simulink model "TosAeb"  (R2021a)
settings: Solver=FixedStepDiscrete  StartTime=0.0  StopTime=inf  FixedStep=0.01  RelTol=1e-3
undefined symbols (not defined in the file or in its folder): ACCModSts, AccSts1, AEB_ObjectMovingOrientation, AEB_ObjectMovingState, CN_BTCActive, CN_CurrentGearR, CN_CurrentGearR_DHT, CN_PTCActive, CN_VDCActive, CN_VSC_EspOff, CN_VSC_FCW_AEB_Error, CN_VSC_FCW_AEB_NoDispay, CN_VSC_FCW_AEB_PerfmDgdtn, CN_VSC_FCW_AEB_TempUnAvlbl, CN_VSC_VehStandstill, CNaccelAvoidMax, CNaccelAvoidMin, CNbrakePedalPress, CNcomfortStrLeftAccelMax, CNcomfortStrLeftAccelMin, CNcomfortStrRightAccelMax, CNcomfortStrRightAccelMin, CNDrvDoorOpen, CNDrvModeEco, CNDrvModeNorm, CNDrvModeOther, CNDrvModeSport, CNDrvSBRreminder, CNNull, CNobjInvalidID, CNPassDoorOpen, CNPassSBRreminder, CNPEBSAccelNegInf, CNPEBSAccelNull, CNPEBSTimeInf, CNPEBSTimeNull, CNSTOPMAX, CNSTOPNULL, CNsubjWidth, CNSwtOFF, CNSwtON, CNTTCMAX, CNTTCNULL, CNVehConfigAEBCycNone, CNVehConfigAEBNone, CNVehConfigAEBPedNone, CNVehConfigFCWNone, CNVehConfigJANone, CollisionSeverity, FALSE, FCWSnvty, JACycObjCalcInfoDefaultParams, JAPedObjCalcInfoDefaultParams, JAVehObjCalcInfoDefaultParams, latCycObjCalcInfoDefaultParams, latPedObjCalcInfoDefaultParams, longObjCalcInfoDefaultParams, ModSts, movingStatus, ObjectType ... (+1112)
libraries: simulink x3031, AEBSFunctionLib x50, Ta_Library x33, AEBSStateMachineLib x23, Mylibrary x12, simulink_extras x7, ObjSelect_lib x3, dspstat3 x2
(part 85 of 102)

== system TosAeb/AEB_Objsel/TA_Processor/AEB_ObjSelect/TargetInfoCheck/Data_precess/TA_EffectCalculate/Obj_FilterFlgCalculate/Long_ObjFilter/OverlapCalculate/BoudingBoxCalculate_Else/Overlap_FlgCalculate/AbsPosn_InsectionFlg/SubjBoundingBox/SubjLUCoordinate ==
  inputSignals : Inport
  Add : Sum  Inputs=++--  OutDataTypeStr=single
  Add1 : Sum  Inputs=+++-  OutDataTypeStr=single
  Bus Selector : BusSelector  OutputSignals=dxPreRefPoint,dxFrontBumperToRearAxis,sincosCoef.cosCoef,subjWidth,sincosCoef.sinCoef,Long_Offset
  Bus Selector1 : BusSelector  OutputSignals=dyPreRefPoint,dxFrontBumperToRearAxis,sincosCoef.sinCoef,subjWidth,sincosCoef.cosCoef,Lat_Offset
  Gain : Gain  Gain=0.5  OutDataTypeStr=single
  Gain1 : Gain  Gain=0.5  OutDataTypeStr=single
  Product{,1..3} (4 alike) : Product  OutDataTypeStr=single
  dxPreLU : Outport
  dyPreLU : Outport  Port=2
  wires:
  Bus Selector/4 -> Product1/1  "<subjWidth>"
  Product3/1 -> Gain1/1
  Bus Selector1/1 -> Add1/1  "<dyPreRefPoint>"
  Gain1/1 -> Add1/3
  Bus Selector1/4 -> Product3/1  "<subjWidth>"
  Product2/1 -> Add1/2
  Bus Selector/3 -> Product/2  "<cosCoef>"
  Add1/1 -> dyPreLU/1
  Bus Selector/5 -> Product1/2  "<sinCoef>"
  Bus Selector/2 -> Product/1  "<dxFrontBumperToRearAxis>"
  Bus Selector1/2 -> Product2/1  "<dxFrontBumperToRearAxis>"
  inputSignals/1 -> Bus Selector1/1, Bus Selector/1
  Bus Selector1/5 -> Product3/2  "<cosCoef>"
  Bus Selector/1 -> Add/1  "<dxPreRefPoint>"
  Gain/1 -> Add/3
  Product1/1 -> Gain/1
  Product/1 -> Add/2
  Bus Selector1/3 -> Product2/2  "<sinCoef>"
  Bus Selector/6 -> Add/4  "<Long_Offset>"
  Add/1 -> dxPreLU/1
  Bus Selector1/6 -> Add1/4  "<Lat_Offset>"

== system TosAeb/AEB_Objsel/TA_Processor/AEB_ObjSelect/TargetInfoCheck/Data_precess/TA_EffectCalculate/Obj_FilterFlgCalculate/Long_ObjFilter/OverlapCalculate/BoudingBoxCalculate_Else/Overlap_FlgCalculate/AbsPosn_InsectionFlg/SubjBoundingBox/SubjRLCoordinate ==
  inputSignals : Inport
  Add6 : Sum  Inputs=+-+-  OutDataTypeStr=single
  Add7 : Sum  Inputs=+---  OutDataTypeStr=single
  Bus Selector6 : BusSelector  OutputSignals=dxPreRefPoint,dxRearBumperToRearAxis,sincosCoef.cosCoef,subjWidth,sincosCoef.sinCoef,Long_Offset
  Bus Selector7 : BusSelector  OutputSignals=dyPreRefPoint,dxRearBumperToRearAxis,sincosCoef.sinCoef,subjWidth,sincosCoef.cosCoef,Lat_Offset
  Gain6 : Gain  Gain=0.5  OutDataTypeStr=single
  Gain7 : Gain  Gain=0.5  OutDataTypeStr=single
  Product{12..15} (4 alike) : Product  OutDataTypeStr=single
  dxPreRL : Outport
  dyPreRL : Outport  Port=2
  wires:
  Product12/1 -> Add6/2
  Gain6/1 -> Add6/3
  Product13/1 -> Gain6/1
  Add6/1 -> dxPreRL/1
  Bus Selector6/4 -> Product13/1  "<subjWidth>"
  Bus Selector6/5 -> Product13/2  "<sinCoef>"
  Bus Selector6/1 -> Add6/1  "<dxPreRefPoint>"
  Bus Selector6/2 -> Product12/1  "<dxRearBumperToRearAxis>"
  Bus Selector6/3 -> Product12/2  "<cosCoef>"
  inputSignals/1 -> Bus Selector7/1, Bus Selector6/1
  Add7/1 -> dyPreRL/1
  Product14/1 -> Add7/2
  Gain7/1 -> Add7/3
  Bus Selector7/1 -> Add7/1  "<dyPreRefPoint>"
  Bus Selector7/2 -> Product14/1  "<dxRearBumperToRearAxis>"
  Bus Selector7/4 -> Product15/1  "<subjWidth>"
  Product15/1 -> Gain7/1
  Bus Selector7/5 -> Product15/2  "<cosCoef>"
  Bus Selector7/3 -> Product14/2  "<sinCoef>"
  Bus Selector6/6 -> Add6/4  "<Long_Offset>"
  Bus Selector7/6 -> Add7/4  "<Lat_Offset>"

== system TosAeb/AEB_Objsel/TA_Processor/AEB_ObjSelect/TargetInfoCheck/Data_precess/TA_EffectCalculate/Obj_FilterFlgCalculate/Long_ObjFilter/OverlapCalculate/BoudingBoxCalculate_Else/Overlap_FlgCalculate/AbsPosn_InsectionFlg/SubjBoundingBox/SubjRUCoordinate ==
  inputSignals : Inport
  Add4 : Sum  Inputs=+++-  OutDataTypeStr=single
  Add5 : Sum  Inputs=++--  OutDataTypeStr=single
  Bus Selector4 : BusSelector  OutputSignals=dxPreRefPoint,dxFrontBumperToRearAxis,sincosCoef.cosCoef,subjWidth,sincosCoef.sinCoef,Long_Offset
  Bus Selector5 : BusSelector  OutputSignals=dyPreRefPoint,dxFrontBumperToRearAxis,sincosCoef.sinCoef,subjWidth,sincosCoef.cosCoef,Lat_Offset
  Gain4 : Gain  Gain=0.5  OutDataTypeStr=single
  Gain5 : Gain  Gain=0.5  OutDataTypeStr=single
  Product{8..11} (4 alike) : Product  OutDataTypeStr=single
  dxPreRU : Outport
  dyPreRU : Outport  Port=2
  wires:
  Bus Selector4/4 -> Product9/1  "<subjWidth>"
  Bus Selector4/5 -> Product9/2  "<sinCoef>"
  Bus Selector4/3 -> Product8/2  "<cosCoef>"
  Bus Selector4/1 -> Add4/1  "<dxPreRefPoint>"
  inputSignals/1 -> Bus Selector5/1, Bus Selector4/1
  Bus Selector4/2 -> Product8/1  "<dxFrontBumperToRearAxis>"
  Product9/1 -> Gain4/1
  Bus Selector5/4 -> Product11/1  "<subjWidth>"
  Product10/1 -> Add5/2
  Product11/1 -> Gain5/1
  Add5/1 -> dyPreRU/1
  Gain5/1 -> Add5/3
  Bus Selector5/2 -> Product10/1  "<dxFrontBumperToRearAxis>"
  Bus Selector5/3 -> Product10/2  "<sinCoef>"
  Bus Selector5/5 -> Product11/2  "<cosCoef>"
  Add4/1 -> dxPreRU/1
  Gain4/1 -> Add4/3
  Product8/1 -> Add4/2
  Bus Selector5/1 -> Add5/1  "<dyPreRefPoint>"
  Bus Selector4/6 -> Add4/4  "<Long_Offset>"
  Bus Selector5/6 -> Add5/4  "<Lat_Offset>"

== system TosAeb/AEB_Objsel/TA_Processor/AEB_ObjSelect/TargetInfoCheck/Data_precess/TA_EffectCalculate/Obj_FilterFlgCalculate/Long_ObjFilter/OverlapCalculate/BoudingBoxCalculate_Else/Overlap_FlgCalculate/AbsPosn_InsectionFlg/SubjBoundingBox/Subsystem ==
  dxFrontBumperToRearAxis : Inport
  sincosCoef : Inport  Port=2
  Bus Selector : BusSelector  OutputSignals=sinCoef,cosCoef
  Product : Product
  Product1 : Product
  Lat_Offset : Outport
  Long_Offset : Outport  Port=2
  wires:
  Product/1 -> Lat_Offset/1  "Lat_Offset"
  dxFrontBumperToRearAxis/1 -> Product1/1, Product/1
  sincosCoef/1 -> Bus Selector/1
  Bus Selector/1 -> Product/2  "<sinCoef>"
  Bus Selector/2 -> Product1/2  "<cosCoef>"
  Product1/1 -> Long_Offset/1  "Long_Offset"

== system TosAeb/AEB_Objsel/TA_Processor/AEB_ObjSelect/TargetInfoCheck/Data_precess/TA_EffectCalculate/Obj_FilterFlgCalculate/Long_ObjFilter/OverlapCalculate/BoudingBoxCalculate_Else/PosnatTTI/LongitudinalMotionData/Subsystem1/Obj_InfoCal ==
  stop : Inport
  TA_ObjAccel : Inport  Port=2
  Subj_Ax : Inport  Port=3
  Subj_Ay : Inport  Port=4
  MappedAngle : Inport  Port=5
  Add : Sum
  Add1 : Sum
  Constant : Constant  Value=0  OutDataTypeStr=single
  Product : Product
  Product1 : Product
  Sin : Trigonometry  Operator=cos
  Sin1 : Trigonometry
  Switch : Switch  Criteria=u2 > Threshold
  Switch1 : Switch  Criteria=u2 > Threshold
  Obj_AbsAcclx : Outport
  Obj_AbsAccly : Outport  Port=2
  Ref_ax : Outport  Port=3
  Ref_ay : Outport  Port=4
  wires:
  Constant/1 -> Switch1/1
  Switch1/1 -> Ref_ay/1
  [Ta_ObjAccl] -> Switch/1
  Switch/1 -> Ref_ax/1
  stop/1 -> Switch1/2, Switch/2
  Add1/1 -> Obj_AbsAccly/1
  Subj_Ay/1 -> Add1/2
  Product1/1 -> Add1/1, Switch1/3
  Sin1/1 -> Product1/1
  [Ta_ObjAccl] -> Product1/2
  Subj_Ax/1 -> Add/2
  Add/1 -> Obj_AbsAcclx/1
  Product/1 -> Add/1, Switch/3
  TA_ObjAccel/1 -> Product/1, [Ta_ObjAccl]
  Sin/1 -> Product/2
  MappedAngle/1 -> Sin/1, Sin1/1

== system TosAeb/AEB_Objsel/TA_Processor/AEB_ObjSelect/TargetInfoCheck/Data_precess/TA_EffectCalculate/Obj_FilterFlgCalculate/Long_ObjFilter/OverlapCalculate/BoudingBoxCalculate_Else/PosnatTTI/LongitudinalMotionData/Subsystem1/Subj_AyCal ==
  Subj_Vx : Inport
  Subj_Vy : Inport  Port=2
  ACC_x : Inport  Port=3
  Add : Sum  Inputs=-+
  Atan2 : Trigonometry  Operator=atan2
  Compare To Constant : simulink/Logic and Bit Operations/Compare To Constant  relop===  const=0  OutDataTypeStr=boolean
  Constant : Constant  Value=pi/2  OutDataTypeStr=single
  Constant1 : Constant  Value=0  OutDataTypeStr=single
  Constant2 : Constant  Value=0.01  OutDataTypeStr=single
  Delay : Delay
  Divide : Product  Inputs=*/
  MapToAngularInterval : Ta_Library/MapToAngularInterval  SystemSampleTime=-1  Latency=0  AutoFrameSizeCalculation=off
  Product : Product
  Sin : Trigonometry  Operator=tan
  Switch : Switch  Criteria=u2 > Threshold
  Terminator : Terminator
  Subj_Ay : Outport
  wires:
  Divide/1 -> Terminator/1
  Constant2/1 -> Divide/2
  Add/1 -> Divide/1
  Delay/1 -> Add/1
  Product/1 -> Subj_Ay/1
  ACC_x/1 -> Product/1
  Switch/1 -> Product/2
  Constant1/1 -> Switch/1
  Compare To Constant/1 -> Switch/2
  Sin/1 -> Compare To Constant/1, Switch/3
  MapToAngularInterval/1 -> Sin/1
  Atan2/1 -> MapToAngularInterval/1
  Constant/1 -> MapToAngularInterval/2
  Subj_Vy/1 -> Atan2/1, Delay/1, Add/2
  Subj_Vx/1 -> Atan2/2

== system TosAeb/AEB_Objsel/TA_Processor/AEB_ObjSelect/TargetInfoCheck/Data_precess/TA_EffectCalculate/Obj_FilterFlgCalculate/Long_ObjFilter/OverlapCalculate/BoudingBoxCalculate_Else/PosnatTTI/PredictHostPositionAtTTR/CalculateHeading/CheckSpeed ==
  note: Threshold for detectable host speed.
  note: 0.2m/s
  x : Inport
  y : Inport  Port=2
  LatSpdThresholdNonVeh1 : Constant  Value=SpdThdInAsyEvlrCritEve
  Product : Product
  Product1 : Product
  Product2 : Product
  Relational Operator : RelationalOperator  Operator=>  OutDataTypeStr=boolean  RndMeth=Nearest
  Subtract : Sum
  SpeedValid : Outport
  wires:
  y/1 -> Product/1, Product/2
  Subtract/1 -> Relational Operator/1  "VelocitySquared"
  Product1/1 -> Subtract/1
  Product/1 -> Subtract/2
  x/1 -> Product1/2, Product1/1
  LatSpdThresholdNonVeh1/1 -> Product2/1, Product2/2
  Product2/1 -> Relational Operator/2
  Relational Operator/1 -> SpeedValid/1  "SpeedValid"

== system TosAeb/AEB_Objsel/TA_Processor/AEB_ObjSelect/TargetInfoCheck/Data_precess/TA_EffectCalculate/Obj_FilterFlgCalculate/Long_ObjFilter/OverlapCalculate/BoudingBoxCalculate_Else/PosnatTTI/PredictHostPositionAtTTR/CalculateHeading/ExtractVelocitiesAtStartOfSegments ==
  PathEstimn : Inport
  Assignment : Assignment  OutputInitialize=Specify size for each dimension in table  IndexOptions=Index vector (port)  Indices=1  OutputSizes=4
  Assignment1 : Assignment  OutputInitialize=Specify size for each dimension in table  IndexOptions=Index vector (port)  Indices=1  OutputSizes=4
  Assignment2 : Assignment  OutputInitialize=Specify size for each dimension in table  IndexOptions=Index vector (port)  Indices=1  OutputSizes=4
  CheckSpeed : Subsystem
  ExtractVelocityVectorFirstPoint : Subsystem
  For Iterator : ForIterator  ResetStates=reset  IterationLimit=4  IterationVariableDataType=int8
  Selector : Selector  InputPortWidth=4  IndexOptions=Index vector (port)  Indices=1  OutputSizes=1
  SpeedValid : Outport
  SegmentVelocitiesX : Outport  Port=2
  SegmentVelocitiesY : Outport  Port=3
  wires:
  Assignment2/1 -> SegmentVelocitiesY/1  "SegmentVelocitiesY"
  Assignment1/1 -> SegmentVelocitiesX/1  "SegmentVelocitiesX"
  Assignment/1 -> SpeedValid/1  "SpeedValid"
  CheckSpeed/1(SpeedValid) -> Assignment/1
  For Iterator/1 -> Selector/2, Assignment1/2, Assignment2/2, Assignment/2
  PathEstimn/1 -> Selector/1
  ExtractVelocityVectorFirstPoint/2(dy) -> Assignment2/1, CheckSpeed/2(y)
  ExtractVelocityVectorFirstPoint/1(dx) -> Assignment1/1, CheckSpeed/1(x)
  Selector/1 -> ExtractVelocityVectorFirstPoint/1(Segment)  "Segment"

== system TosAeb/AEB_Objsel/TA_Processor/AEB_ObjSelect/TargetInfoCheck/Data_precess/TA_EffectCalculate/Obj_FilterFlgCalculate/Long_ObjFilter/OverlapCalculate/BoudingBoxCalculate_Else/PosnatTTI/PredictHostPositionAtTTR/SegmentComponents/SafeDivide ==
  Numerator : Inport  OutDataTypeStr=DataType
      settings: AttributesFormatString=DT=%<OutDataTypeStr>
  Denominator : Inport  Port=2  OutDataTypeStr=DataType
      settings: AttributesFormatString=DT=%<OutDataTypeStr>
  SafeDivide : MATLAB Function
  Quotient : Outport  OutDataTypeStr=DataType
      settings: AttributesFormatString=DT=%<OutDataTypeStr>
  wires:
  SafeDivide/1(Quotient) -> Quotient/1
  Denominator/1 -> SafeDivide/2(Denominator)  "Denominator"
  Numerator/1 -> SafeDivide/1(Numerator)  "Numerator"

== system TosAeb/AEB_Objsel/TA_Processor/AEB_ObjSelect/TargetInfoCheck/Data_precess/TA_EffectCalculate/Obj_FilterFlgCalculate/Long_ObjFilter/OverlapCalculate/BoudingBoxCalculate_Else/PosnatTTI/PredictHostPositionAtTTR/SelectSegment/ForEachSegmentInPahEstimn ==
  PahEstimn : Inport  OutDataTypeStr=Bus: PahEstimn
  Time : Inport  Port=2
  Assignment1 : Assignment  OutputInitialize=Specify size for each dimension in table  IndexOptions=Index vector (port)  Indices=1  OutputSizes=4
  Bus Selector : BusSelector  OutputSignals=TiAry
  For Iterator : ForIterator  ResetStates=reset  IterationSource=external  IterationVariableDataType=int8
      settings: AttributesFormatString=DT=%<IterationVariableDataType>\nResetStates=%<ResetStates>
  Logical Operator : Logic  AllPortsSameDT=off  OutDataTypeStr=boolean
  Min : MinMax  Function=max  OutDataTypeStr=single
  Min1 : MinMax  OutDataTypeStr=single
  Relational Operator : RelationalOperator  Operator=>  OutDataTypeStr=boolean  RndMeth=Nearest  InputSameDT=on
      settings: AttributesFormatString=InputSameDT=%<InputSameDT>
  Relational Operator1 : RelationalOperator  Operator=<=  OutDataTypeStr=boolean  RndMeth=Nearest  InputSameDT=on
      settings: AttributesFormatString=InputSameDT=%<InputSameDT>
  Selector2 : Selector  IndexOptions=Index vector (port)  Indices=[1 3]  OutputSizes=1
  Width : Width  DataType=int8
  TimeWithinSegment : Outport
  wires:
  Min/1 -> Relational Operator1/2
  Bus Selector/1 -> Min/1, Min1/1  "<TiAry>"
  Time/1 -> Relational Operator1/1, [Time]
  Min1/1 -> Relational Operator/2
  [Time] -> Relational Operator/1
  Relational Operator1/1 -> Logical Operator/1
  Relational Operator/1 -> Logical Operator/2
  [CurrentIndex] -> Selector2/2
  [CurrentIndex] -> Assignment1/2
  Logical Operator/1 -> Assignment1/1  "TimeWithinSegment"
  Assignment1/1 -> TimeWithinSegment/1
  PahEstimn/1 -> Selector2/1, [PahEstimn]
  Selector2/1 -> Bus Selector/1
  Width/1 -> For Iterator/1
  [PahEstimn] -> Width/1
  For Iterator/1 -> [CurrentIndex]

== system TosAeb/AEB_Objsel/TA_Processor/AEB_ObjSelect/TargetInfoCheck/Data_precess/TA_EffectCalculate/Obj_FilterFlgCalculate/Long_ObjFilter/TTR_Calculate/CalculateTTRAndTTM_else/LongitudinalTTRComputer/CalculateTTI/HostAndObjectMoving/CheckIfSolutionExists ==
  note: 相对加速度
  note: 相对速度
  note: This subsystem checks if there is a solution to the motion equation ARel*t^2/2 + VRel*t + EdgeDistance = 0.
      |
      | The cases with positive and negative ARel are handled separately.
      | If ARel == 0, there is always a solution if VRel ~= 0.
      | The case VRel == 0 and ARel == 0 is handled outside this subsystem.
  note: Same zero threshold as in the
      | quadratic equation solver.
  note: Solution exists if ARel == 0.
  EdgeDistance : Inport
  VRel : Inport  Port=2
  ARel : Inport  Port=3
  Compare To Zero : Subsystem  mask(Compare To Zero){relop=>, OutDataTypeStr=boolean, ZeroCross=on}
      mask prompts: relop "Simulink:masks:Operator_MP"; OutDataTypeStr "Simulink:masks:OutputDataType_MP"; ZeroCross "Simulink:masks:EnableZerocrossingDetection_MP"
      mask help: Simulink:masks:CompareToZero_MD
      mask init: same as TosAeb/AEB_Objsel/TA_Processor/AEB_ObjSelect/TargetInfoCheck/Data_precess/TA_EffectCalculate/Obj_FilterFlgCalculate/Long_ObjFilter/TTR_Calculate/CalculateTTRAndTTM_else/ModifyTTRAtSameSpeedAndAcceleration/Compare To Zero
      mask TypeOptions relop: == | ~= | < | <= | >= | >
      mask TypeOptions OutDataTypeStr: Simulink:masks:uint8 | Simulink:masks:boolean
      mask Callback ZeroCross: sl('zeroCrossEnable', gcbh);
      mask Display: disp([relop,' 0'])
  Compare To Zero1 : Subsystem  mask(Compare To Zero){relop=<, OutDataTypeStr=boolean, ZeroCross=on}
      mask prompts: relop "Simulink:masks:Operator_MP"; OutDataTypeStr "Simulink:masks:OutputDataType_MP"; ZeroCross "Simulink:masks:EnableZerocrossingDetection_MP"
      mask help: Simulink:masks:CompareToZero_MD
      mask init: same as TosAeb/AEB_Objsel/TA_Processor/AEB_ObjSelect/TargetInfoCheck/Data_precess/TA_EffectCalculate/Obj_FilterFlgCalculate/Long_ObjFilter/TTR_Calculate/CalculateTTRAndTTM_else/ModifyTTRAtSameSpeedAndAcceleration/Compare To Zero
      mask TypeOptions relop: == | ~= | < | <= | >= | >
      mask TypeOptions OutDataTypeStr: Simulink:masks:uint8 | Simulink:masks:boolean
      mask Callback ZeroCross: sl('zeroCrossEnable', gcbh);
      mask Display: disp([relop,' 0'])
  Constant : Constant  Value=false  OutDataTypeStr=boolean
  Gain : Gain  Gain=2
  LimitedSafeDivide : Subsystem
  Logop_1 : Logic  AllPortsSameDT=off  OutDataTypeStr=boolean
  Logop_2 : Logic  AllPortsSameDT=off  OutDataTypeStr=boolean
  Logop_3 : Logic  Operator=OR  AllPortsSameDT=off  OutDataTypeStr=boolean
  Relop_1 : RelationalOperator  Operator=>  OutDataTypeStr=boolean  RndMeth=Nearest
  Relop_2 : RelationalOperator  Operator=<  OutDataTypeStr=boolean  RndMeth=Nearest
  SizeChecker : Subsystem  mask(Size checker){UseInput=off, Limit=1e-2, DataTypeIn=single, Operator=>}
      mask prompts: UseInput "Take the limit as an input"; DataTypeIn "Data type:"; Operator "Relational operator:"
      mask help: The block tests the absolute value of the supplied signal against a specified value using the selected operator. If the signal conforms to the test, true is returned; else, false is returned. The limit can either be set in the mask or as an external signal.
      mask init: same as TosAeb/AEB_Objsel/TA_Processor/AEB_ObjSelect/TargetInfoCheck/Data_precess/TA_EffectCalculate/Obj_FilterFlgCalculate/Long_ObjFilter/TTR_Calculate/CalculateTTRAndTTM_else/ModifyTTRAtSameSpeedAndAcceleration/SizeChecker
      settings: AttributesFormatString=DT=%<DataTypeIn>
      mask Help: The block tests the absolute value of the supplied signal against a specified value using the selected operator. If the signal conforms to the test, true is returned; else, false is returned. The limit can either be set in the mask or as an external signal.
      mask Callback UseInput:
      | % Author: Jakob Andersson (jande573)
      | 
      | % Enable and disable the box for the limit variable depending on the status of the checkbox
      | if strcmp(get_param(gcb,'UseInput'),'on')
      |     % Disable the box
      |     set_param(gcb,'MaskEnables',{'on','off','on','on'}); % One setting for each parameter in this mask; needs to be updated if the parameters are changed
      | else
      |     % Enable the box
      |     set_param(gcb,'MaskEnables',{'on','on','on','on'}); % One setting for each parameter in this mask; needs to be updated if the parameters are changed
      | end
      mask Callback Limit:
      | % Author: Jakob Andersson (jande573)
      | 
      | % Define the default value to apply if the entered value is disallowed
      | default='1e-6';
      | 
      | % Evaluate the entered value
      | val=eval(get_param(gcb,'Limit'));
      | 
      | % Test the entered value and revert to the default if necessary
      | if isnumeric(val)==false
      |     % Set the default if the entered value did not evaluate to a numeric
      |     set_param(gcb,'Limit',default);
      |     errordlg('The limit must be numeric. The default value will be used instead.');
      | elseif val<0
      |     % Set the default if the entered value is negative
      |     set_param(gcb,'Limit',default);
      |     errordlg('The limit must be positive since it is compared to an absolute value. The default value will be used instead.');
      | end
      mask TypeOptions Operator:
      | Relational
      | Operator/Operator
      mask Callback Operator:
      | % Author: Jakob Andersson (jande573)
      | 
      | % Define the default value to apply if the entered value is disallowed
      | EnabledOperators={'==','~=','<','<=','>=','>'};
      | default='>';
      | 
      | % Evaluate the selected operator and determine if it is ok
      | ok=any(strcmp(get_param(gcb,'Operator'),EnabledOperators));
      | 
      | % Revert the operator if it is disabled
      | if ~ok
      |     % Set the default if the entered value did not evaluate to a numeric
      |     set_param(gcb,'Operator',default);
      |     errordlg('The selected operator is disabled. The default operator will be used instead.');
      | end
      mask Display:
      | % Display the mask for this block
      | % Author: Jakob Andersson (jande573)
      | 
      | % Transform the operator to TeX
      | switch Operator
      |     case '=='
      |         op='=';
      |     case '~='
      |         op='\neq';
      |     case '<='
      |         op='\leq';
      |     case '>='
      |         op='\geq';
      |     otherwise
      |         op=Operator;
      | end
      | 
      | % Display the correct mask depending on the number of inputs
      | if strcmp(UseInput,'on')
      |     % Display the block logic and the set limit
      |     disp(horzcat('|u| ',op,' a'),'texmode','on');
      | 
      |     % Display the port title
      |     port_label('input',1,'u');
      |     port_label('input',2,'a');
      | else
      |     % Display the block logic and the set limit
      |     disp(horzcat('|u| ',op,' ',num2str(Limit)),'texmode','on');
      | 
      |     % Display the port title
      |     port_label('input',1,'u');
      | end
  Square : Math  Operator=square  SignedPower=on  SaturateOnIntegerOverflow=on
  Switch : Switch  Criteria=u2 ~= 0
  NoSolution : Outport
  wires:
  Constant/1 -> Switch/3  "False"
  Switch/1 -> NoSolution/1  "NoSolution"
  SizeChecker/1(TestOk) -> Switch/2  "ARelIsDetectable"
  VRel/1 -> Square/1
  ARel/1 -> Compare To Zero/1(u), Compare To Zero1/1(u), SizeChecker/1(Signal), Gain/1
  EdgeDistance/1 -> Relop_1/1, Relop_2/1
  Square/1 -> LimitedSafeDivide/1(Numerator)
  Gain/1 -> LimitedSafeDivide/2(Denominator)
  LimitedSafeDivide/1(LimitedQuotient) -> Relop_1/2, Relop_2/2
  Compare To Zero/1(y) -> Logop_1/2  "PositiveARel"
  Relop_1/1 -> Logop_1/1
  Compare To Zero1/1(y) -> Logop_2/2  "NegativeARel"
  Logop_1/1 -> Logop_3/1  "NoSolutionPositiveARel"
  Logop_2/1 -> Logop_3/2  "NoSolutionNegativeARel"
  Logop_3/1 -> Switch/1  "NoSolutionCalculatedUsingARel"
  [BelowLimit] -> Logop_2/1
  Relop_2/1 -> [BelowLimit]

== system TosAeb/AEB_Objsel/TA_Processor/AEB_ObjSelect/TargetInfoCheck/Data_precess/TA_EffectCalculate/Obj_FilterFlgCalculate/Long_ObjFilter/TTR_Calculate/CalculateTTRAndTTM_else/LongitudinalTTRComputer/CalculateTTI/HostAndObjectMoving/CheckIfSolutionExists1 ==
  note: This subsystem checks if there is a solution to the motion equation ARel*t^2/2 + VRel*t + EdgeDistance = 0.
      |
      | The cases with positive and negative ARel are handled separately.
      | If ARel == 0, there is always a solution if VRel ~= 0.
      | The case VRel == 0 and ARel == 0 is handled outside this subsystem.
  note: Same zero threshold as in the
      | quadratic equation solver.
  note: Solution exists if ARel == 0.
  EdgeDistance : Inport
  VRel : Inport  Port=2
  ARel : Inport  Port=3
  Compare To Zero : Subsystem  mask(Compare To Zero){relop=>, OutDataTypeStr=boolean, ZeroCross=on}
      mask prompts: relop "Simulink:masks:Operator_MP"; OutDataTypeStr "Simulink:masks:OutputDataType_MP"; ZeroCross "Simulink:masks:EnableZerocrossingDetection_MP"
      mask help: Simulink:masks:CompareToZero_MD
      mask init: same as TosAeb/AEB_Objsel/TA_Processor/AEB_ObjSelect/TargetInfoCheck/Data_precess/TA_EffectCalculate/Obj_FilterFlgCalculate/Long_ObjFilter/TTR_Calculate/CalculateTTRAndTTM_else/ModifyTTRAtSameSpeedAndAcceleration/Compare To Zero
      mask TypeOptions relop: == | ~= | < | <= | >= | >
      mask TypeOptions OutDataTypeStr: Simulink:masks:uint8 | Simulink:masks:boolean
      mask Callback ZeroCross: sl('zeroCrossEnable', gcbh);
      mask Display: disp([relop,' 0'])
  Compare To Zero1 : Subsystem  mask(Compare To Zero){relop=<, OutDataTypeStr=boolean, ZeroCross=on}
      mask prompts: relop "Simulink:masks:Operator_MP"; OutDataTypeStr "Simulink:masks:OutputDataType_MP"; ZeroCross "Simulink:masks:EnableZerocrossingDetection_MP"
      mask help: Simulink:masks:CompareToZero_MD
      mask init: same as TosAeb/AEB_Objsel/TA_Processor/AEB_ObjSelect/TargetInfoCheck/Data_precess/TA_EffectCalculate/Obj_FilterFlgCalculate/Long_ObjFilter/TTR_Calculate/CalculateTTRAndTTM_else/ModifyTTRAtSameSpeedAndAcceleration/Compare To Zero
      mask TypeOptions relop: == | ~= | < | <= | >= | >
      mask TypeOptions OutDataTypeStr: Simulink:masks:uint8 | Simulink:masks:boolean
      mask Callback ZeroCross: sl('zeroCrossEnable', gcbh);
      mask Display: disp([relop,' 0'])
  Constant : Constant  Value=false  OutDataTypeStr=boolean
  Gain : Gain  Gain=2
  LimitedSafeDivide : Subsystem
  Logop_1 : Logic  AllPortsSameDT=off  OutDataTypeStr=boolean
  Logop_2 : Logic  AllPortsSameDT=off  OutDataTypeStr=boolean
  Logop_3 : Logic  Operator=OR  AllPortsSameDT=off  OutDataTypeStr=boolean
  Relop_1 : RelationalOperator  Operator=>  OutDataTypeStr=boolean  RndMeth=Nearest
  Relop_2 : RelationalOperator  Operator=<  OutDataTypeStr=boolean  RndMeth=Nearest
  SizeChecker : Subsystem  mask(Size checker){UseInput=off, Limit=1e-2, DataTypeIn=single, Operator=>}
      mask prompts: UseInput "Take the limit as an input"; DataTypeIn "Data type:"; Operator "Relational operator:"
      mask help: The block tests the absolute value of the supplied signal against a specified value using the selected operator. If the signal conforms to the test, true is returned; else, false is returned. The limit can either be set in the mask or as an external signal.
      mask init: same as TosAeb/AEB_Objsel/TA_Processor/AEB_ObjSelect/TargetInfoCheck/Data_precess/TA_EffectCalculate/Obj_FilterFlgCalculate/Long_ObjFilter/TTR_Calculate/CalculateTTRAndTTM_else/ModifyTTRAtSameSpeedAndAcceleration/SizeChecker
      settings: AttributesFormatString=DT=%<DataTypeIn>
      mask Help: The block tests the absolute value of the supplied signal against a specified value using the selected operator. If the signal conforms to the test, true is returned; else, false is returned. The limit can either be set in the mask or as an external signal.
      mask Callback UseInput:
      | % Author: Jakob Andersson (jande573)
      | 
      | % Enable and disable the box for the limit variable depending on the status of the checkbox
      | if strcmp(get_param(gcb,'UseInput'),'on')
      |     % Disable the box
      |     set_param(gcb,'MaskEnables',{'on','off','on','on'}); % One setting for each parameter in this mask; needs to be updated if the parameters are changed
      | else
      |     % Enable the box
      |     set_param(gcb,'MaskEnables',{'on','on','on','on'}); % One setting for each parameter in this mask; needs to be updated if the parameters are changed
      | end
      mask Callback Limit:
      | % Author: Jakob Andersson (jande573)
      | 
      | % Define the default value to apply if the entered value is disallowed
      | default='1e-6';
      | 
      | % Evaluate the entered value
      | val=eval(get_param(gcb,'Limit'));
      | 
      | % Test the entered value and revert to the default if necessary
      | if isnumeric(val)==false
      |     % Set the default if the entered value did not evaluate to a numeric
      |     set_param(gcb,'Limit',default);
      |     errordlg('The limit must be numeric. The default value will be used instead.');
      | elseif val<0
      |     % Set the default if the entered value is negative
      |     set_param(gcb,'Limit',default);
      |     errordlg('The limit must be positive since it is compared to an absolute value. The default value will be used instead.');
      | end
      mask TypeOptions Operator:
      | Relational
      | Operator/Operator
      mask Callback Operator:
      | % Author: Jakob Andersson (jande573)
      | 
      | % Define the default value to apply if the entered value is disallowed
      | EnabledOperators={'==','~=','<','<=','>=','>'};
      | default='>';
      | 
      | % Evaluate the selected operator and determine if it is ok
      | ok=any(strcmp(get_param(gcb,'Operator'),EnabledOperators));
      | 
      | % Revert the operator if it is disabled
      | if ~ok
      |     % Set the default if the entered value did not evaluate to a numeric
      |     set_param(gcb,'Operator',default);
      |     errordlg('The selected operator is disabled. The default operator will be used instead.');
      | end
      mask Display:
      | % Display the mask for this block
      | % Author: Jakob Andersson (jande573)
      | 
      | % Transform the operator to TeX
      | switch Operator
      |     case '=='
      |         op='=';
      |     case '~='
      |         op='\neq';
      |     case '<='
      |         op='\leq';
      |     case '>='
      |         op='\geq';
      |     otherwise
      |         op=Operator;
      | end
      | 
      | % Display the correct mask depending on the number of inputs
      | if strcmp(UseInput,'on')
      |     % Display the block logic and the set limit
      |     disp(horzcat('|u| ',op,' a'),'texmode','on');
      | 
      |     % Display the port title
      |     port_label('input',1,'u');
      |     port_label('input',2,'a');
      | else
      |     % Display the block logic and the set limit
      |     disp(horzcat('|u| ',op,' ',num2str(Limit)),'texmode','on');
      | 
      |     % Display the port title
      |     port_label('input',1,'u');
      | end
  Square : Math  Operator=square  SignedPower=on  SaturateOnIntegerOverflow=on
  Switch : Switch  Criteria=u2 ~= 0
  NoSolution : Outport
  wires:
  Constant/1 -> Switch/3  "False"
  Switch/1 -> NoSolution/1  "NoSolution"
  SizeChecker/1(TestOk) -> Switch/2  "ARelIsDetectable"
  VRel/1 -> Square/1
  ARel/1 -> Compare To Zero/1(u), Compare To Zero1/1(u), SizeChecker/1(Signal), Gain/1
  EdgeDistance/1 -> Relop_1/1, Relop_2/1
  Square/1 -> LimitedSafeDivide/1(Numerator)
  Gain/1 -> LimitedSafeDivide/2(Denominator)
  LimitedSafeDivide/1(LimitedQuotient) -> Relop_1/2, Relop_2/2
  Compare To Zero/1(y) -> Logop_1/2  "PositiveARel"
  Relop_1/1 -> Logop_1/1
  Compare To Zero1/1(y) -> Logop_2/2  "NegativeARel"
  Logop_1/1 -> Logop_3/1  "NoSolutionPositiveARel"
  Logop_2/1 -> Logop_3/2  "NoSolutionNegativeARel"
  Logop_3/1 -> Switch/1  "NoSolutionCalculatedUsingARel"
  [BelowLimit] -> Logop_2/1
  Relop_2/1 -> [BelowLimit]

== system TosAeb/AEB_Objsel/TA_Processor/AEB_ObjSelect/TargetInfoCheck/Data_precess/TA_EffectCalculate/Obj_FilterFlgCalculate/Long_ObjFilter/TTR_Calculate/CalculateTTRAndTTM_else/LongitudinalTTRComputer/CalculateTTI/HostAndObjectMoving/TimeToIntersectionComputer ==
  note: 求解一元二次方程，并判断是否存在解、
      | 依据位置关系进行判断输出TTI数值
  note: 100
  note: -100
  note: a*t^2/2 + v*t + x = 0
  VRel : Inport  OutDataTypeStr=single
      settings: AttributesFormatString=DT=%<OutDataTypeStr>
  ARel : Inport  Port=2  OutDataTypeStr=single
      settings: AttributesFormatString=DT=%<OutDataTypeStr>
  Posn : Inport  Port=3  OutDataTypeStr=single
      settings: AttributesFormatString=DT=%<OutDataTypeStr>
  AEB_State : Inport  Port=4
  Abs : Abs
  Abs1 : Abs
  Compare To Constant : simulink/Logic and Bit Operations/Compare To Constant  relop=<=  const=0.25  OutDataTypeStr=boolean
  Compare To Constant1 : simulink/Logic and Bit Operations/Compare To Constant  relop===  const=0  OutDataTypeStr=boolean
  Compare To Constant10 : simulink/Logic and Bit Operations/Compare To Constant  relop===  const=0  OutDataTypeStr=boolean
  Compare To Constant2 : simulink/Logic and Bit Operations/Compare To Constant  relop=~=  const=0  OutDataTypeStr=boolean
  Compare To Constant3 : simulink/Logic and Bit Operations/Compare To Constant  relop=<=  const=0.2  OutDataTypeStr=boolean
  Compare To Constant4 : simulink/Logic and Bit Operations/Compare To Constant  relop=~=  const=100  OutDataTypeStr=boolean
  Compare To Constant5 : simulink/Logic and Bit Operations/Compare To Constant  relop=~=  const=-100  OutDataTypeStr=boolean
  Compare To Constant7 : simulink/Logic and Bit Operations/Compare To Constant  relop=~=  const=0  OutDataTypeStr=boolean
  Compare To Constant8 : simulink/Logic and Bit Operations/Compare To Constant  relop=<=  const=6  OutDataTypeStr=boolean
  Compare To Constant9 : simulink/Logic and Bit Operations/Compare To Constant  relop=<=  const=6  OutDataTypeStr=boolean
  Compare To Zero : Subsystem  mask(Compare To Zero){relop=<, OutDataTypeStr=boolean, ZeroCross=on}
      mask prompts: relop "Simulink:masks:Operator_MP"; OutDataTypeStr "Simulink:masks:OutputDataType_MP"; ZeroCross "Simulink:masks:EnableZerocrossingDetection_MP"
      mask help: Simulink:masks:CompareToZero_MD
      mask init: same as TosAeb/AEB_Objsel/TA_Processor/AEB_ObjSelect/TargetInfoCheck/Data_precess/TA_EffectCalculate/Obj_FilterFlgCalculate/Long_ObjFilter/TTR_Calculate/CalculateTTRAndTTM_else/ModifyTTRAtSameSpeedAndAcceleration/Compare To Zero
      mask TypeOptions relop: == | ~= | < | <= | >= | >
      mask TypeOptions OutDataTypeStr: Simulink:masks:uint8 | Simulink:masks:boolean
      mask Callback ZeroCross: sl('zeroCrossEnable', gcbh);
      mask Display: disp([relop,' 0'])
  Constant : Constant  OutDataTypeStr=single
  Constant1 : Constant  Value=P_TA_TiLowrLimInAsyEvlrCritEve  OutDataTypeStr=single
      settings: AttributesFormatString=DT=%<OutDataTypeStr>
  Constant3 : Constant  Value=P_TA_TiUpprLimInAsyEvlrCritEve  OutDataTypeStr=single
      settings: AttributesFormatString=DT=%<OutDataTypeStr>
  Delay : Delay  DelayLength=5
  Divide : Product  Inputs=*/
  Gain : Gain  Gain=0.5
  Logical Operator : Logic  Operator=OR  Inputs=3  AllPortsSameDT=off  OutDataTypeStr=boolean
  Logical Operator1 : Logic  Inputs=3  AllPortsSameDT=off  OutDataTypeStr=boolean
  Logical Operator2 : Logic  AllPortsSameDT=off  OutDataTypeStr=boolean
  Logical Operator3 : Logic  Inputs=3  AllPortsSameDT=off  OutDataTypeStr=boolean
  QuadraticEquationSolver : MATLAB Function  TreatAsAtomicUnit=on  RTWFileName=QuadraticEquationSolverNew  mask(Quadratic equation solver){ZeroThreshold=single(1e-2)}
      mask prompts: ZeroThreshold "Zero threshold:"
      mask help:
      | This block solves a quadratic polynomial equation.
      | Both solutions are returned in a bus.
      | If the equation lacks real roots then zeros are returned,
      | along with a flag indicating that no solutions where found.
      settings: RTWFcnNameOpts=Use subsystem name
  Relational Operator : RelationalOperator  Operator=<=  OutDataTypeStr=boolean  RndMeth=Simplest
  SolutionChooser : Subsystem
  Switch : Switch  Criteria=u2 > Threshold
  Switch1 : Switch  Criteria=u2 ~= 0  InputSameDT=on
      settings: AttributesFormatString=InputSameDT=%<InputSameDT>
  Switch2 : Switch  Criteria=u2 ~= 0  InputSameDT=on
      settings: AttributesFormatString=InputSameDT=%<InputSameDT>
  Switch3 : Switch  Criteria=u2 > Threshold
  Switch4 : Switch  Criteria=u2 > Threshold
  Terminator1 : Terminator
  Unit Delay : UnitDelay  SampleTime=-1  HasFrameUpgradeWarning=on
  TimeToIntersection : Outport  OutDataTypeStr=single
      settings: AttributesFormatString=DT=%<OutDataTypeStr>
  wires:
  Switch1/1 -> Switch2/3  "DefaultTTI"
  Constant1/1 -> Switch1/1  "DefaultNegativeTTI"
  Compare To Zero/1(y) -> Switch1/2
  SolutionChooser/1(Solution) -> Switch2/1
  QuadraticEquationSolver/2(SolutionFound) -> [Solution], Switch2/2
  QuadraticEquationSolver/1(x) -> SolutionChooser/1(Solutions)
  Posn/1 -> [B], Divide/1, QuadraticEquationSolver/3(c), Compare To Zero/1(u)  "Posn"
  VRel/1 -> QuadraticEquationSolver/2(b), [VRel]  "VRel"
  Switch2/1 -> Switch/3  "TimeToIntersection"
  Constant3/1 -> Switch1/3  "DefaultPositiveTTI"
  Gain/1 -> QuadraticEquationSolver/1(a)  "ARelHalf"
  [ARel] -> SolutionChooser/2(ARel)
  [ARel] -> Abs1/1
  [Solution] -> Compare To Constant1/1
  Compare To Constant1/1 -> Logical Operator/2
  Compare To Constant/1 -> Logical Operator/1
  Compare To Constant3/1 -> Switch3/2
  Constant/1 -> Switch3/1
  Switch3/1 -> Divide/2
  Abs/1 -> Switch3/3, Compare To Constant3/1
  Divide/1 -> [A]
  [A] -> Switch/1
  Switch4/1 -> Unit Delay/1, TimeToIntersection/1
  Abs1/1 -> Compare To Constant/1
  Unit Delay/1 -> Compare To Constant8/1, Compare To Constant5/1, Compare To Constant4/1, Switch4/1
  [A] -> Compare To Constant9/1, Compare To Constant7/1
  [B] -> Delay/1, Relational Operator/1
  Compare To Constant4/1 -> Logical Operator1/1
  Compare To Constant5/1 -> Logical Operator1/2
  Compare To Constant8/1 -> Logical Operator1/3
  [Solution] -> Compare To Constant10/1
  Delay/1 -> Relational Operator/2
  Compare To Constant7/1 -> Logical Operator2/1
  Compare To Constant9/1 -> Logical Operator2/2
  Logical Operator1/1 -> Logical Operator3/1
  Logical Operator2/1 -> Logical Operator3/2
  Relational Operator/1 -> Terminator1/1
  Logical Operator3/1 -> Switch4/2
  Switch/1 -> Switch4/3
  Compare To Constant10/1 -> Logical Operator3/3
  Logical Operator/1 -> Switch/2
  AEB_State/1 -> Compare To Constant2/1
  Compare To Constant2/1 -> Logical Operator/3
  [VRel] -> Abs/1
  ARel/1 -> [ARel], Gain/1  "ARel"

== system TosAeb/AEB_Objsel/TA_Processor/AEB_ObjSelect/TargetInfoCheck/Data_precess/TA_EffectCalculate/Obj_FilterFlgCalculate/Long_ObjFilter/TTR_Calculate/CalculateTTRAndTTM_else/LongitudinalTTRComputer/CalculateTTI/HostAndObjectMoving/TimeToIntersectionComputer1 ==
  note: a*t^2/2 + v*t + x = 0
  note: -100
  note: 100
  note: 求解一元二次方程，并判断是否存在解、
      | 依据位置关系进行判断输出TTI数值
  VRel : Inport  OutDataTypeStr=single
      settings: AttributesFormatString=DT=%<OutDataTypeStr>
  ARel : Inport  Port=2  OutDataTypeStr=single
      settings: AttributesFormatString=DT=%<OutDataTypeStr>
  Posn : Inport  Port=3  OutDataTypeStr=single
      settings: AttributesFormatString=DT=%<OutDataTypeStr>
  Abs : Abs
  Abs1 : Abs
  Compare To Constant : simulink/Logic and Bit Operations/Compare To Constant  relop=<=  const=0.25  OutDataTypeStr=boolean
  Compare To Constant1 : simulink/Logic and Bit Operations/Compare To Constant  relop===  const=0  OutDataTypeStr=boolean
  Compare To Constant10 : simulink/Logic and Bit Operations/Compare To Constant  relop===  const=0  OutDataTypeStr=boolean
  Compare To Constant2 : simulink/Logic and Bit Operations/Compare To Constant  relop=<  const=0  OutDataTypeStr=boolean
  Compare To Constant3 : simulink/Logic and Bit Operations/Compare To Constant  relop===  const=0  OutDataTypeStr=boolean
  Compare To Constant4 : simulink/Logic and Bit Operations/Compare To Constant  relop=~=  const=100  OutDataTypeStr=boolean
  Compare To Constant5 : simulink/Logic and Bit Operations/Compare To Constant  relop=~=  const=-100  OutDataTypeStr=boolean
  Compare To Constant7 : simulink/Logic and Bit Operations/Compare To Constant  relop=~=  const=0  OutDataTypeStr=boolean
  Compare To Constant8 : simulink/Logic and Bit Operations/Compare To Constant  relop=<=  const=6  OutDataTypeStr=boolean
  Compare To Constant9 : simulink/Logic and Bit Operations/Compare To Constant  relop=<=  const=6  OutDataTypeStr=boolean
  Compare To Zero : Subsystem  mask(Compare To Zero){relop=<, OutDataTypeStr=boolean, ZeroCross=on}
      mask prompts: relop "Simulink:masks:Operator_MP"; OutDataTypeStr "Simulink:masks:OutputDataType_MP"; ZeroCross "Simulink:masks:EnableZerocrossingDetection_MP"
      mask help: Simulink:masks:CompareToZero_MD
      mask init: same as TosAeb/AEB_Objsel/TA_Processor/AEB_ObjSelect/TargetInfoCheck/Data_precess/TA_EffectCalculate/Obj_FilterFlgCalculate/Long_ObjFilter/TTR_Calculate/CalculateTTRAndTTM_else/ModifyTTRAtSameSpeedAndAcceleration/Compare To Zero
      mask TypeOptions relop: == | ~= | < | <= | >= | >
      mask TypeOptions OutDataTypeStr: Simulink:masks:uint8 | Simulink:masks:boolean
      mask Callback ZeroCross: sl('zeroCrossEnable', gcbh);
      mask Display: disp([relop,' 0'])
  Constant : Constant  OutDataTypeStr=single
  Constant1 : Constant  Value=P_TA_TiLowrLimInAsyEvlrCritEve  OutDataTypeStr=single
      settings: AttributesFormatString=DT=%<OutDataTypeStr>
  Constant3 : Constant  Value=P_TA_TiUpprLimInAsyEvlrCritEve  OutDataTypeStr=single
      settings: AttributesFormatString=DT=%<OutDataTypeStr>
  Delay : Delay  DelayLength=5
  Divide : Product  Inputs=*/
  Gain : Gain  Gain=0.5
  Logical Operator : Logic  Inputs=3  AllPortsSameDT=off  OutDataTypeStr=boolean
  Logical Operator1 : Logic  Inputs=3  AllPortsSameDT=off  OutDataTypeStr=boolean
  Logical Operator2 : Logic  AllPortsSameDT=off  OutDataTypeStr=boolean
  Logical Operator3 : Logic  Inputs=4  AllPortsSameDT=off  OutDataTypeStr=boolean
  QuadraticEquationSolver : MATLAB Function  TreatAsAtomicUnit=on  RTWFileName=QuadraticEquationSolverNew  mask(Quadratic equation solver){ZeroThreshold=single(1e-2)}
      mask prompts: ZeroThreshold "Zero threshold:"
      mask help:
      | This block solves a quadratic polynomial equation.
      | Both solutions are returned in a bus.
      | If the equation lacks real roots then zeros are returned,
      | along with a flag indicating that no solutions where found.
      settings: RTWFcnNameOpts=Use subsystem name
  Relational Operator : RelationalOperator  Operator=<=  OutDataTypeStr=boolean  RndMeth=Simplest
  SolutionChooser : Subsystem
  Subsystem : Subsystem
  Switch : Switch  Criteria=u2 > Threshold
  Switch1 : Switch  Criteria=u2 ~= 0  InputSameDT=on
      settings: AttributesFormatString=InputSameDT=%<InputSameDT>
  Switch2 : Switch  Criteria=u2 ~= 0  InputSameDT=on
      settings: AttributesFormatString=InputSameDT=%<InputSameDT>
  Switch3 : Switch  Criteria=u2 > Threshold
  Switch4 : Switch  Criteria=u2 > Threshold
  Unit Delay : UnitDelay  SampleTime=-1  HasFrameUpgradeWarning=on
  TimeToIntersection : Outport  OutDataTypeStr=single
      settings: AttributesFormatString=DT=%<OutDataTypeStr>
  wires:
  Compare To Constant10/1 -> Logical Operator3/4
  Switch/1 -> Switch4/3
  Logical Operator3/1 -> Switch4/2
  Relational Operator/1 -> Logical Operator3/3
  Logical Operator2/1 -> Logical Operator3/2
  Logical Operator1/1 -> Logical Operator3/1
  Compare To Constant9/1 -> Logical Operator2/2
  Compare To Constant7/1 -> Logical Operator2/1
  Delay/1 -> Relational Operator/2
  [Solution] -> Compare To Constant10/1
  Compare To Constant8/1 -> Logical Operator1/3
  Compare To Constant5/1 -> Logical Operator1/2
  Compare To Constant4/1 -> Logical Operator1/1
  [B] -> Relational Operator/1, Delay/1
  [A] -> Compare To Constant7/1, Compare To Constant9/1
  Unit Delay/1 -> Switch4/1, Compare To Constant4/1, Compare To Constant5/1, Compare To Constant8/1
  ARel/1 -> Subsystem/1(ax)  "ARel"
  Subsystem/1(Out1) -> Gain/1, [ARel]  "ARel"
  Abs1/1 -> Compare To Constant/1
  Switch4/1 -> Unit Delay/1, TimeToIntersection/1
  [A] -> Switch/1
  Divide/1 -> [A]
  Abs/1 -> Switch3/3
  Switch3/1 -> Divide/2
  [VRel] -> Compare To Constant3/1, Abs/1
  Constant/1 -> Switch3/1
  Compare To Constant3/1 -> Switch3/2
  Compare To Constant2/1 -> Logical Operator/3
  [VRel] -> Compare To Constant2/1
  Logical Operator/1 -> Switch/2
  Compare To Constant1/1 -> Logical Operator/2
  Compare To Constant/1 -> Logical Operator/1
  [Solution] -> Compare To Constant1/1
  [ARel] -> Abs1/1
  [ARel] -> SolutionChooser/2(ARel)
  Gain/1 -> QuadraticEquationSolver/1(a)  "ARelHalf"
  Constant3/1 -> Switch1/3  "DefaultPositiveTTI"
  Switch2/1 -> Switch/3  "TimeToIntersection"
  VRel/1 -> [VRel], QuadraticEquationSolver/2(b)  "VRel"
  Posn/1 -> Compare To Zero/1(u), QuadraticEquationSolver/3(c), Divide/1, [B]  "Posn"
  QuadraticEquationSolver/1(x) -> SolutionChooser/1(Solutions)
  QuadraticEquationSolver/2(SolutionFound) -> Switch2/2, [Solution]
  SolutionChooser/1(Solution) -> Switch2/1
  Compare To Zero/1(y) -> Switch1/2
  Constant1/1 -> Switch1/1  "DefaultNegativeTTI"
  Switch1/1 -> Switch2/3  "DefaultTTI"
